simulink library "ee_lib_for_power_systems"  (R2025b)
settings: Solver=VariableStepAuto  StartTime=0.0  StopTime=10.0  RelTol=1e-3
undefined symbols (not defined in the file or in its folder): Alt_PolePair_Count, C3m, DiscIntNeverNeededParam, Enum1to12, L3m, Total_SM_Inertia, Ts
libraries: fl_lib x291, nesl_utility x219, ee_lib x139, custom_lib x12, $bdroot x10, ee_sl_lib x3, eeTransforms x2, eeGeneralControl x1, simulink x1
(part 8 of 11)

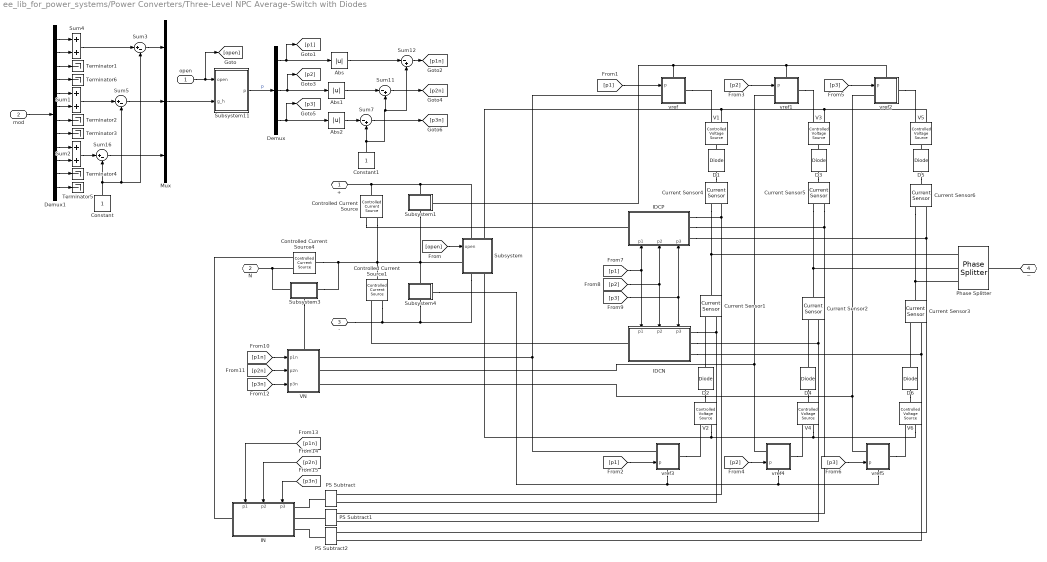
== system ee_lib_for_power_systems/Power Converters/Three-Level NPC Average-Switch with Diodes ==
  open : Inport
  mod : Inport  Port=2
  Abs : Abs  SaturateOnIntegerOverflow=off
  Abs1 : Abs  SaturateOnIntegerOverflow=off
  Abs2 : Abs  SaturateOnIntegerOverflow=off
  Constant : Constant  SampleTime=Ts
  Constant1 : Constant  SampleTime=Ts
  Controlled Current Source : fl_lib/Electrical/Electrical Sources/Controlled Current Source
  Controlled Current Source1 : fl_lib/Electrical/Electrical Sources/Controlled Current Source
  Controlled Current Source4 : fl_lib/Electrical/Electrical Sources/Controlled Current Source
  Current Sensor{1..6} (6 alike) : fl_lib/Electrical/Electrical Sensors/Current Sensor
  D{1..6} (6 alike) : fl_lib/Electrical/Electrical Elements/Diode  Vf=Vf V  Ron=Ron Ohm  Goff=Goff 1/Ohm  i_specify=off  i_priority=None  i=0 A  i_nominal_specify=off  i_nominal_value=1  i_nominal_unit=A  v_specify=off  v_priority=None  v=0 V  v_nominal_specify=off  v_nominal_value=1  v_nominal_unit=V
  Demux : Demux  Outputs=3
  Demux1 : Demux  Outputs=12
  IDCN : Subsystem
      settings: PortSchema="{\"entries\":[{\"content\":{\"sides\":[{\"content\":{\"connectorIds\":[\"LConn1\",\"LConn2\",\"LConn3\"]},\"type\":\"ConnectorPlacement.EquallySpacedRectSide\",\"uuid\":\"5fd9aafb-b495-44e2-9499-8cdfe2ab4f56\"},{\"content\":{\"connectorIds\":[\"RConn1\"],\"side\":\"RIGHT\"},\"type\":\"ConnectorPlacement.EquallySpacedRectSide\",\"uuid\":\"0d8ceae3-09aa-4016-97de-ee2249771a91\"},{\"content\":{\"connectorIds\":[\"In1\",\"In2\",\"In3\"],\"side\":\"TOP\"},\"type\":\"ConnectorPlacement.EquallySpacedRectSide\",\"uuid\":\"0b4c8ea8-b524-451d-8365-47bbe880e547\"},{\"content\":{\"side\":\"BOTTOM\"},\"type\":\"ConnectorPlacement.EquallySpacedRectSide\",\"uuid\":\"91c10802-5411-4afb-b6a0-ea8a924d9d4e\"}]},\"type\":\"ConnectorPlacement.EquallySpacedConnector\",\"uuid\":\"44c8dc65-a8bd-4e02-863f-9a87b0ac0f96\"}],\"packageUris\":[\"http://schema.mathworks.com/mf0/Connector/R2020b\"],\"version\":\"1.0\"}"
  IDCP : Subsystem
      settings: PortSchema="{\"entries\":[{\"content\":{\"sides\":[{\"content\":{\"connectorIds\":[\"LConn1\",\"LConn2\",\"LConn3\"]},\"type\":\"ConnectorPlacement.EquallySpacedRectSide\",\"uuid\":\"5fd9aafb-b495-44e2-9499-8cdfe2ab4f56\"},{\"content\":{\"connectorIds\":[\"RConn1\"],\"side\":\"RIGHT\"},\"type\":\"ConnectorPlacement.EquallySpacedRectSide\",\"uuid\":\"0d8ceae3-09aa-4016-97de-ee2249771a91\"},{\"content\":{\"side\":\"TOP\"},\"type\":\"ConnectorPlacement.EquallySpacedRectSide\",\"uuid\":\"0b4c8ea8-b524-451d-8365-47bbe880e547\"},{\"content\":{\"connectorIds\":[\"In1\",\"In2\",\"In3\"],\"side\":\"BOTTOM\"},\"type\":\"ConnectorPlacement.EquallySpacedRectSide\",\"uuid\":\"91c10802-5411-4afb-b6a0-ea8a924d9d4e\"}]},\"type\":\"ConnectorPlacement.EquallySpacedConnector\",\"uuid\":\"44c8dc65-a8bd-4e02-863f-9a87b0ac0f96\"}],\"packageUris\":[\"http://schema.mathworks.com/mf0/Connector/R2020b\"],\"version\":\"1.0\"}"
  IN : Subsystem
      settings: PortSchema="{\"entries\":[{\"content\":{\"sides\":[{\"content\":{\"connectorIds\":[\"LConn1\",\"LConn2\",\"LConn3\"]},\"type\":\"ConnectorPlacement.EquallySpacedRectSide\",\"uuid\":\"5fd9aafb-b495-44e2-9499-8cdfe2ab4f56\"},{\"content\":{\"connectorIds\":[\"RConn1\"],\"side\":\"RIGHT\"},\"type\":\"ConnectorPlacement.EquallySpacedRectSide\",\"uuid\":\"0d8ceae3-09aa-4016-97de-ee2249771a91\"},{\"content\":{\"connectorIds\":[\"In1\",\"In2\",\"In3\"],\"side\":\"TOP\"},\"type\":\"ConnectorPlacement.EquallySpacedRectSide\",\"uuid\":\"0b4c8ea8-b524-451d-8365-47bbe880e547\"},{\"content\":{\"side\":\"BOTTOM\"},\"type\":\"ConnectorPlacement.EquallySpacedRectSide\",\"uuid\":\"91c10802-5411-4afb-b6a0-ea8a924d9d4e\"}]},\"type\":\"ConnectorPlacement.EquallySpacedConnector\",\"uuid\":\"44c8dc65-a8bd-4e02-863f-9a87b0ac0f96\"}],\"packageUris\":[\"http://schema.mathworks.com/mf0/Connector/R2020b\"],\"version\":\"1.0\"}"
  Mux : Mux  Inputs=3
  PS Subtract : fl_lib/Physical Signals/Functions/PS Subtract
  PS Subtract1 : fl_lib/Physical Signals/Functions/PS Subtract
  PS Subtract2 : fl_lib/Physical Signals/Functions/PS Subtract
  Phase Splitter : ee_lib/Connectors & References/Phase Splitter
  Subsystem : Subsystem
      settings: PortSchema="{\"entries\":[{\"content\":{\"sides\":[{\"content\":{\"connectorIds\":[\"In1\",\"LConn1\"]},\"type\":\"ConnectorPlacement.EquallySpacedRectSide\",\"uuid\":\"88db1bd8-f64b-4165-8d2c-7cfa4014cba2\"},{\"content\":{\"side\":\"RIGHT\"},\"type\":\"ConnectorPlacement.EquallySpacedRectSide\",\"uuid\":\"19f0c499-9eed-48d3-a8f5-fe9dad581b85\"},{\"content\":{\"connectorIds\":[\"LConn2\",\"LConn3\"],\"side\":\"TOP\"},\"type\":\"ConnectorPlacement.EquallySpacedRectSide\",\"uuid\":\"71d5fe89-ffc2-4430-819d-512bfd197a1c\"},{\"content\":{\"connectorIds\":[\"RConn1\",\"RConn2\"],\"side\":\"BOTTOM\"},\"type\":\"ConnectorPlacement.EquallySpacedRectSide\",\"uuid\":\"b828cb58-5f78-4b23-b8a0-147c7a9d81e7\"}]},\"type\":\"ConnectorPlacement.EquallySpacedConnector\",\"uuid\":\"64b174d4-80a0-4eb7-9a75-e3677787263f\"}],\"packageUris\":[\"http://schema.mathworks.com/mf0/Connector/R2020b\"],\"version\":\"1.0\"}"
  Subsystem1 : Subsystem
      settings: PortSchema="{\"entries\":[{\"content\":{\"sides\":[{\"content\":{\"connectorIds\":[\"LConn1\"]},\"type\":\"ConnectorPlacement.EquallySpacedRectSide\",\"uuid\":\"657010cc-ebac-44a1-b1fa-70bc77d723fd\"},{\"content\":{\"connectorIds\":[\"RConn1\"],\"side\":\"RIGHT\"},\"type\":\"ConnectorPlacement.EquallySpacedRectSide\",\"uuid\":\"4e06f12d-4c30-449d-8090-9eefcb844bcd\"},{\"content\":{\"side\":\"TOP\"},\"type\":\"ConnectorPlacement.EquallySpacedRectSide\",\"uuid\":\"366a080c-b244-4111-b523-cca255cda3b4\"},{\"content\":{\"connectorIds\":[\"RConn2\"],\"side\":\"BOTTOM\"},\"type\":\"ConnectorPlacement.EquallySpacedRectSide\",\"uuid\":\"d10f8831-4450-471d-a877-48a3f6294e5e\"}]},\"type\":\"ConnectorPlacement.EquallySpacedConnector\",\"uuid\":\"eaac196a-9b15-4081-b7ac-14663ddf5688\"}],\"packageUris\":[\"http://schema.mathworks.com/mf0/Connector/R2020b\"],\"version\":\"1.0\"}"
  Subsystem11 : Subsystem
  Subsystem3 : Subsystem
      settings: PortSchema="{\"entries\":[{\"content\":{\"sides\":[{\"content\":{\"connectorIds\":[\"LConn1\"]},\"type\":\"ConnectorPlacement.EquallySpacedRectSide\",\"uuid\":\"657010cc-ebac-44a1-b1fa-70bc77d723fd\"},{\"content\":{\"connectorIds\":[\"RConn1\"],\"side\":\"RIGHT\"},\"type\":\"ConnectorPlacement.EquallySpacedRectSide\",\"uuid\":\"4e06f12d-4c30-449d-8090-9eefcb844bcd\"},{\"content\":{\"connectorIds\":[\"LConn2\"],\"side\":\"TOP\"},\"type\":\"ConnectorPlacement.EquallySpacedRectSide\",\"uuid\":\"366a080c-b244-4111-b523-cca255cda3b4\"},{\"content\":{\"side\":\"BOTTOM\"},\"type\":\"ConnectorPlacement.EquallySpacedRectSide\",\"uuid\":\"d10f8831-4450-471d-a877-48a3f6294e5e\"}]},\"type\":\"ConnectorPlacement.EquallySpacedConnector\",\"uuid\":\"eaac196a-9b15-4081-b7ac-14663ddf5688\"}],\"packageUris\":[\"http://schema.mathworks.com/mf0/Connector/R2020b\"],\"version\":\"1.0\"}"
  Subsystem4 : Subsystem
      settings: PortSchema="{\"entries\":[{\"content\":{\"sides\":[{\"content\":{\"connectorIds\":[\"LConn1\"]},\"type\":\"ConnectorPlacement.EquallySpacedRectSide\",\"uuid\":\"657010cc-ebac-44a1-b1fa-70bc77d723fd\"},{\"content\":{\"connectorIds\":[\"RConn1\"],\"side\":\"RIGHT\"},\"type\":\"ConnectorPlacement.EquallySpacedRectSide\",\"uuid\":\"4e06f12d-4c30-449d-8090-9eefcb844bcd\"},{\"content\":{\"side\":\"TOP\"},\"type\":\"ConnectorPlacement.EquallySpacedRectSide\",\"uuid\":\"366a080c-b244-4111-b523-cca255cda3b4\"},{\"content\":{\"connectorIds\":[\"RConn2\"],\"side\":\"BOTTOM\"},\"type\":\"ConnectorPlacement.EquallySpacedRectSide\",\"uuid\":\"d10f8831-4450-471d-a877-48a3f6294e5e\"}]},\"type\":\"ConnectorPlacement.EquallySpacedConnector\",\"uuid\":\"eaac196a-9b15-4081-b7ac-14663ddf5688\"}],\"packageUris\":[\"http://schema.mathworks.com/mf0/Connector/R2020b\"],\"version\":\"1.0\"}"
  Sum1 : Sum
  Sum11 : Sum  Inputs=|-+
  Sum12 : Sum  Inputs=|-+
  Sum16 : Sum  Inputs=|+-
  Sum2 : Sum
  Sum3 : Sum  Inputs=|+-
  Sum4 : Sum
  Sum5 : Sum  Inputs=|+-
  Sum7 : Sum  Inputs=|-+
  Terminator{1..6} (6 alike) : Terminator
  V{1..6} (6 alike) : fl_lib/Electrical/Electrical Sources/Controlled Voltage Source
  VN : Subsystem
      settings: PortSchema="{\"entries\":[{\"content\":{\"sides\":[{\"content\":{\"connectorIds\":[\"In1\",\"In2\",\"In3\"]},\"type\":\"ConnectorPlacement.EquallySpacedRectSide\",\"uuid\":\"76446245-3b58-4011-85fd-f27f8ae281c8\"},{\"content\":{\"connectorIds\":[\"RConn1\",\"RConn2\",\"RConn3\"],\"side\":\"RIGHT\"},\"type\":\"ConnectorPlacement.EquallySpacedRectSide\",\"uuid\":\"0729b849-5916-4866-a3f0-df0ddf2f6c39\"},{\"content\":{\"connectorIds\":[\"LConn1\"],\"side\":\"TOP\"},\"type\":\"ConnectorPlacement.EquallySpacedRectSide\",\"uuid\":\"732bc8a4-0f08-4f07-8caa-b1f4c499d2f5\"}]},\"type\":\"ConnectorPlacement.EquallySpacedConnector\",\"uuid\":\"056633d8-005f-41b2-9737-b2dcd7b802cb\"}],\"packageUris\":[\"http://schema.mathworks.com/mf0/Connector/R2020b\"],\"version\":\"1.0\"}"
  vref : Subsystem
      settings: PortSchema="{\"entries\":[{\"content\":{\"sides\":[{\"content\":{\"connectorIds\":[\"In1\",\"LConn1\"]},\"type\":\"ConnectorPlacement.EquallySpacedRectSide\",\"uuid\":\"177b0f26-b09e-4dc8-aee9-3547e6e38c36\"},{\"content\":{\"connectorIds\":[\"RConn1\"],\"side\":\"RIGHT\"},\"type\":\"ConnectorPlacement.EquallySpacedRectSide\",\"uuid\":\"51253abf-338b-44bf-b091-8aed177abf09\"},{\"content\":{\"connectorIds\":[\"LConn2\"],\"side\":\"TOP\"},\"type\":\"ConnectorPlacement.EquallySpacedRectSide\",\"uuid\":\"62148f0b-b6fd-4066-b7ab-63aa80b90d1b\"}]},\"type\":\"ConnectorPlacement.EquallySpacedConnector\",\"uuid\":\"16fdcc32-ca93-497b-869b-72fbbd5f95c5\"}],\"packageUris\":[\"http://schema.mathworks.com/mf0/Connector/R2020b\"],\"version\":\"1.0\"}"
  vref1 : Subsystem
      settings: PortSchema="{\"entries\":[{\"content\":{\"sides\":[{\"content\":{\"connectorIds\":[\"In1\",\"LConn1\"]},\"type\":\"ConnectorPlacement.EquallySpacedRectSide\",\"uuid\":\"177b0f26-b09e-4dc8-aee9-3547e6e38c36\"},{\"content\":{\"connectorIds\":[\"RConn1\"],\"side\":\"RIGHT\"},\"type\":\"ConnectorPlacement.EquallySpacedRectSide\",\"uuid\":\"51253abf-338b-44bf-b091-8aed177abf09\"},{\"content\":{\"connectorIds\":[\"LConn2\"],\"side\":\"TOP\"},\"type\":\"ConnectorPlacement.EquallySpacedRectSide\",\"uuid\":\"62148f0b-b6fd-4066-b7ab-63aa80b90d1b\"}]},\"type\":\"ConnectorPlacement.EquallySpacedConnector\",\"uuid\":\"16fdcc32-ca93-497b-869b-72fbbd5f95c5\"}],\"packageUris\":[\"http://schema.mathworks.com/mf0/Connector/R2020b\"],\"version\":\"1.0\"}"
  vref2 : Subsystem
      settings: PortSchema="{\"entries\":[{\"content\":{\"sides\":[{\"content\":{\"connectorIds\":[\"In1\",\"LConn1\"]},\"type\":\"ConnectorPlacement.EquallySpacedRectSide\",\"uuid\":\"177b0f26-b09e-4dc8-aee9-3547e6e38c36\"},{\"content\":{\"connectorIds\":[\"RConn1\"],\"side\":\"RIGHT\"},\"type\":\"ConnectorPlacement.EquallySpacedRectSide\",\"uuid\":\"51253abf-338b-44bf-b091-8aed177abf09\"},{\"content\":{\"connectorIds\":[\"LConn2\"],\"side\":\"TOP\"},\"type\":\"ConnectorPlacement.EquallySpacedRectSide\",\"uuid\":\"62148f0b-b6fd-4066-b7ab-63aa80b90d1b\"}]},\"type\":\"ConnectorPlacement.EquallySpacedConnector\",\"uuid\":\"16fdcc32-ca93-497b-869b-72fbbd5f95c5\"}],\"packageUris\":[\"http://schema.mathworks.com/mf0/Connector/R2020b\"],\"version\":\"1.0\"}"
  vref3 : Subsystem
      settings: PortSchema="{\"entries\":[{\"content\":{\"sides\":[{\"content\":{\"connectorIds\":[\"LConn1\",\"In1\"]},\"type\":\"ConnectorPlacement.EquallySpacedRectSide\",\"uuid\":\"177b0f26-b09e-4dc8-aee9-3547e6e38c36\"},{\"content\":{\"connectorIds\":[\"RConn1\"],\"side\":\"RIGHT\"},\"type\":\"ConnectorPlacement.EquallySpacedRectSide\",\"uuid\":\"51253abf-338b-44bf-b091-8aed177abf09\"},{\"content\":{\"side\":\"TOP\"},\"type\":\"ConnectorPlacement.EquallySpacedRectSide\",\"uuid\":\"62148f0b-b6fd-4066-b7ab-63aa80b90d1b\"},{\"content\":{\"connectorIds\":[\"RConn2\"],\"side\":\"BOTTOM\"},\"type\":\"ConnectorPlacement.EquallySpacedRectSide\",\"uuid\":\"f032becc-e905-4cd9-aa70-19abd7f3ae13\"}]},\"type\":\"ConnectorPlacement.EquallySpacedConnector\",\"uuid\":\"16fdcc32-ca93-497b-869b-72fbbd5f95c5\"}],\"packageUris\":[\"http://schema.mathworks.com/mf0/Connector/R2020b\"],\"version\":\"1.0\"}"
  vref4 : Subsystem
      settings: PortSchema="{\"entries\":[{\"content\":{\"sides\":[{\"content\":{\"connectorIds\":[\"LConn1\",\"In1\"]},\"type\":\"ConnectorPlacement.EquallySpacedRectSide\",\"uuid\":\"177b0f26-b09e-4dc8-aee9-3547e6e38c36\"},{\"content\":{\"connectorIds\":[\"RConn1\"],\"side\":\"RIGHT\"},\"type\":\"ConnectorPlacement.EquallySpacedRectSide\",\"uuid\":\"51253abf-338b-44bf-b091-8aed177abf09\"},{\"content\":{\"side\":\"TOP\"},\"type\":\"ConnectorPlacement.EquallySpacedRectSide\",\"uuid\":\"62148f0b-b6fd-4066-b7ab-63aa80b90d1b\"},{\"content\":{\"connectorIds\":[\"RConn2\"],\"side\":\"BOTTOM\"},\"type\":\"ConnectorPlacement.EquallySpacedRectSide\",\"uuid\":\"f032becc-e905-4cd9-aa70-19abd7f3ae13\"}]},\"type\":\"ConnectorPlacement.EquallySpacedConnector\",\"uuid\":\"16fdcc32-ca93-497b-869b-72fbbd5f95c5\"}],\"packageUris\":[\"http://schema.mathworks.com/mf0/Connector/R2020b\"],\"version\":\"1.0\"}"
  vref5 : Subsystem
      settings: PortSchema="{\"entries\":[{\"content\":{\"sides\":[{\"content\":{\"connectorIds\":[\"LConn1\",\"In1\"]},\"type\":\"ConnectorPlacement.EquallySpacedRectSide\",\"uuid\":\"177b0f26-b09e-4dc8-aee9-3547e6e38c36\"},{\"content\":{\"connectorIds\":[\"RConn1\"],\"side\":\"RIGHT\"},\"type\":\"ConnectorPlacement.EquallySpacedRectSide\",\"uuid\":\"51253abf-338b-44bf-b091-8aed177abf09\"},{\"content\":{\"side\":\"TOP\"},\"type\":\"ConnectorPlacement.EquallySpacedRectSide\",\"uuid\":\"62148f0b-b6fd-4066-b7ab-63aa80b90d1b\"},{\"content\":{\"connectorIds\":[\"RConn2\"],\"side\":\"BOTTOM\"},\"type\":\"ConnectorPlacement.EquallySpacedRectSide\",\"uuid\":\"f032becc-e905-4cd9-aa70-19abd7f3ae13\"}]},\"type\":\"ConnectorPlacement.EquallySpacedConnector\",\"uuid\":\"16fdcc32-ca93-497b-869b-72fbbd5f95c5\"}],\"packageUris\":[\"http://schema.mathworks.com/mf0/Connector/R2020b\"],\"version\":\"1.0\"}"
  + : PMIOPort  Side=Left
  N : PMIOPort  Port=2  Side=Left
  - : PMIOPort  Port=3  Side=Left
  ~ : PMIOPort  Port=4  Side=Right
  wires:
  [p3] -> IDCP/1(p3), IDCN/1(p3)
  [p1] -> IDCP/3(p1), IDCN/3(p1)
  Abs2/1 -> Sum7/1
  Abs1/1 -> Sum11/1
  Abs/1 -> Sum12/1
  Constant1/1 -> Sum12/2, Sum11/2, Sum7/2
  Demux/3 -> Abs2/1, [p3]
  Demux/2 -> Abs1/1, [p2]
  Demux1/6 -> Sum1/2
  Sum3/1 -> Mux/1
  Demux1/10 -> Sum2/2
  Demux1/2 -> Sum4/2
  Demux1/9 -> Sum2/1
  Demux1/5 -> Sum1/1
  Sum4/1 -> Sum3/1
  Demux1/1 -> Sum4/1
  [p2] -> vref4/1(p)
  mod/1 -> Demux1/1
  Sum1/1 -> Sum5/1
  Sum2/1 -> Sum16/1
  Sum16/1 -> Mux/3
  Constant/1 -> Sum3/2, Sum5/2, Sum16/2
  [p2] -> IDCP/2(p2), IDCN/2(p2)
  Demux1/7 -> Terminator2/1
  Demux1/8 -> Terminator3/1
  Demux1/11 -> Terminator4/1
  Demux1/12 -> Terminator5/1
  Demux1/3 -> Terminator1/1
  Demux1/4 -> Terminator6/1
  Sum5/1 -> Mux/2
  Demux/1 -> Abs/1, [p1]
  Mux/1 -> Subsystem11/2(g_h)
  Subsystem11/1(p) -> Demux/1  "p"
  open/1 -> Subsystem11/1(open), [open]
  [open] -> Subsystem/1(open)
  Sum12/1 -> [p1n]
  Sum11/1 -> [p2n]
  Sum7/1 -> [p3n]
  [p1n] -> IN/3(p1)
  [p2n] -> IN/2(p2)
  [p3n] -> IN/1(p3)
  [p1] -> vref/1(p)
  [p2] -> vref1/1(p)
  [p3] -> vref2/1(p)
  [p1n] -> VN/1(p1n)
  [p2n] -> VN/2(p2n)
  [p3n] -> VN/3(p3n)
  [p1] -> vref3/1(p)
  [p3] -> vref5/1(p)
  connections:
  D5/RConn1 -- V5/LConn1
  D3/RConn1 -- V3/LConn1
  IDCN/LConn3 -- Current Sensor3/RConn1 -- PS Subtract2/LConn2
  IDCN/LConn2 -- Current Sensor2/RConn1 -- PS Subtract1/LConn2
  IDCN/LConn1 -- Current Sensor1/RConn1 -- PS Subtract/LConn2
  D6/RConn1 -- Current Sensor3/RConn2
  D6/LConn1 -- V6/LConn1
  D4/LConn1 -- V4/LConn1
  D4/RConn1 -- Current Sensor2/RConn2
  Current Sensor4/RConn2 -- Phase Splitter/RConn1 -- Current Sensor1/LConn1
  D2/LConn1 -- V2/LConn1
  Current Sensor4/RConn1 -- PS Subtract/LConn1 -- IDCP/LConn1
  Current Sensor5/RConn1 -- IDCP/LConn2 -- PS Subtract1/LConn1
  IDCP/LConn3 -- Current Sensor6/RConn1 -- PS Subtract2/LConn1
  ~/RConn1 -- Phase Splitter/LConn1
  Current Sensor6/RConn2 -- Phase Splitter/RConn3 -- Current Sensor3/LConn1
  Subsystem/LConn2 -- Subsystem1/LConn1 -- Controlled Current Source/LConn1 -- +/RConn1
  Controlled Current Source/RConn1 -- IDCP/RConn1
  IDCN/RConn1 -- Controlled Current Source1/RConn1
  Subsystem4/RConn2 -- vref5/RConn2 -- vref4/RConn2 -- vref3/RConn2
  D2/RConn1 -- Current Sensor1/RConn2
  vref/LConn2 -- Subsystem1/RConn2 -- vref2/LConn2 -- vref1/LConn2
  Controlled Current Source4/RConn2 -- Subsystem3/LConn1 -- N/RConn1
  V1/RConn2 -- Subsystem/LConn3 -- V3/RConn2 -- V5/RConn2
  D1/RConn1 -- V1/LConn1
  Controlled Current Source1/RConn2 -- -/RConn1 -- Subsystem/RConn1 -- Subsystem4/RConn1
  D1/LConn1 -- Current Sensor4/LConn1
  D3/LConn1 -- Current Sensor5/LConn1
  D5/LConn1 -- Current Sensor6/LConn1
  Current Sensor5/RConn2 -- Phase Splitter/RConn2 -- Current Sensor2/LConn1
  Controlled Current Source4/LConn1 -- Subsystem3/RConn1 -- Controlled Current Source/RConn2 -- Controlled Current Source1/LConn1 -- Subsystem4/LConn1 -- Subsystem1/RConn1 -- Subsystem/LConn1
  vref1/RConn1 -- V3/RConn1
  vref/RConn1 -- V1/RConn1
  vref2/RConn1 -- V5/RConn1
  vref/LConn1 -- vref3/LConn1 -- VN/RConn1
  vref3/RConn1 -- V2/RConn1
  vref4/RConn1 -- V4/RConn1
  VN/RConn2 -- vref4/LConn1 -- vref1/LConn1
  vref5/RConn1 -- V6/RConn1
  VN/RConn3 -- vref5/LConn1 -- vref2/LConn1
  IN/RConn1 -- Controlled Current Source4/RConn1
  PS Subtract/RConn1 -- IN/LConn1
  PS Subtract1/RConn1 -- IN/LConn2
  PS Subtract2/RConn1 -- IN/LConn3
  V6/RConn2 -- V2/RConn2 -- Subsystem/RConn2 -- V4/RConn2
  Subsystem3/LConn2 -- VN/LConn1

== system ee_lib_for_power_systems/Power Converters/Thyristor ==
  g : Inport
  Simulink-PS Converter1 : nesl_utility/Simulink-PS Converter  PhysicalDomain=network_engine_domain  SubClassName=ps_input  LeftPortType=input  RightPortType=output  PseudoPeriodic=off  Frequencies=[]  NoiseDistribution=none  NoiseParameters=[]  Unit=1  AffineConversion=off  FilteringAndDerivatives=zero  SimscapeFilterOrder=1  InputFilterTimeConstant=0.001  UdotUserProvided=0
  thyristor : ee_lib/Semiconductors & Converters/Thyristor (Piecewise Linear)  port_option=ee.enum.controlport.ps  Vf=Vf V  Ron=Ron Ohm  Goff=Goff 1/Ohm  Vgt=0.5 V  Ih=1e-3 A  diode_param=ee.enum.converters.protectiondiode.none  diode_Vf=0.8 V  diode_Ron=0.001 Ohm  diode_Goff=1e-5 1/Ohm  diode_C=50e-9 F  diode_iRM=-235 A  diode_iF=300 A  diode_idot=-50 A/us  diode_trr_param=ee.enum.converters.diodetrr.trr_factor  diode_trr_factor=3  diode_trr=15 us  diode_Qrr=1500 s*uA  iak_specify=off  iak_priority=None  iak=0 A  iak_nominal_specify=off  iak_nominal_value=1  iak_nominal_unit=A  vak_specify=off  vak_priority=None  vak=0 V  vak_nominal_specify=off  vak_nominal_value=1  vak_nominal_unit=V  vgk_specify=off  vgk_priority=None  vgk=0 V  vgk_nominal_specify=off  vgk_nominal_value=1  vgk_nominal_unit=V  thermal_loss_option=ee.enum.converters.thermalLossOption.constantvalues  Von_data=[0, .1, .6, .8, 1, 1.3, 1.6, 2, 2.4; 0, .1, .7, 1, 1.2, 1.5, 1.9, 2.4, 2.8] V  Tj=[298.15, 398.15] K  Iout_data=[0, .1, 1, 5, 10, 20, 40, 70, 100] A  Eon_data=[0, .0024, .024, .12, .2, .48, 1.04, 2.16, 3.24; 0, .003, .03, .15, .25, .6, 1.3, 2.7, 4.05] * 1e-3 J  Tj_loss=[298.15, 398.15] K  Iout_data_loss=[0, .1, 1, 5, 10, 20, 40, 70, 100] A  Eon_scalar=22.86e-3 J  Eoff_natural_commutation=10e-3 J  Voff=300 V  Iout_scalar=600 A  onCurrentMeasDelay=1e-4 s  diodeModelType=ee.enum.converters.diodeModelType.pwl  diodeTableType=ee.enum.diode.tableType.IfMat  diode_IfMat=[.07, .12, .19, 1.75, 4.24, 7.32, 11.2; .16, .3, .72, 2.14, 4.02, 6.35, 9.12] A  diode_VfMat=[.9, 1.15, 1.25, 1.5, 1.75, 2.17, 2.6, 2.85; .58, .68, .75, 1.1, 1.38, 1.77, 2.27, 2.7] V  diode_TjVec=[25, 125] degC  diode_VfVec=[.5, .7, .9, 1.3, 1.7, 2.1, 2.5] V  diode_IfVec=[.1, .2, .5, 1, 2, 4, 7, 10] A  diode_rec_loss_model=ee.enum.diode.reverseRecoveryModel.fixed  diode_ErecVal=0 J  diode_scaleWithIandV=true  diode_ErecMat=zeros(2, 3) J  diode_TjrecVec=[298.15, 398.15] K  diode_IfrecVec=[.1, 1, 10] A  diode_IrecVal=10 A  diode_VrecVal=10 V  diode_tFilter=1 / 1000 / 100 s  thermal_network_parameterization=ee.enum.thermalNetworkTopology.external  Rth=[.08, .5] K/W  Rth_Cauer=[.08, .1, .5] K/W  Rth_Foster=[.08, .14, .22, .16] K/W  thermal_mass_parameterization=ee.enum.thermalNetwork.timeconstant  thermal_mass=[.01, .5] J/K  thermal_mass_Cauer=[.01, .1, .5] J/K  thermal_mass_Foster=[.001, .005, .05, .5] J/K  thermal_time_constant=[.001, .2] s  thermal_time_constant_Cauer=[.001, .1, .2] s  thermal_time_constant_Foster=[7e-05, .0007, .01, .08] s  thermal_mass_initial_temperatures=[25, 25] degC  thermal_mass_initial_temperatures_Cauer=[25, 25, 25] degC  junction_thermal_mass=0.01 J/K  Tvec_initial_Foster=[25, 25, 25, 25] degC  junction_temperature_specify=off  junction_temperature_priority=None  junction_temperature=25 degC  junction_temperature_nominal_specify=off  junction_temperature_nominal_value=1  junction_temperature_nominal_unit=degC  Qj_specify=off  Qj_priority=None  Qj=0 W  Qj_nominal_specify=off  Qj_nominal_value=1  Qj_nominal_unit=W  accumulatedSwitchingLosses_specify=off  accumulatedSwitchingLosses_priority=None  accumulatedSwitchingLosses=0 J
      settings: InternalSimscapePortConfiguration="{\"Bottom\":[],\"Left\":[{\"id\":\"G\",\"label\":\"\",\"type\":\"input\"}],\"Right\":[{\"id\":\"A\",\"label\":\"\",\"type\":\"foundation.electrical.electrical\"},{\"id\":\"K\",\"label\":\"\",\"type\":\"foundation.electrical.electrical\"}],\"Top\":[]}"
  a : PMIOPort  Side=Left
  k : PMIOPort  Port=2  Side=Right
  wires:
  g/1 -> Simulink-PS Converter1/1
  connections:
  thyristor/LConn1 -- Simulink-PS Converter1/RConn1
  thyristor/RConn1 -- a/RConn1
  thyristor/RConn2 -- k/RConn1

== system ee_lib_for_power_systems/Power Converters/Thyristor Bridge (Ideal) ==
  g : Inport
  Demux : Demux  Outputs=6
  Phase Splitter : ee_lib/Connectors & References/Phase Splitter
  S1 : Subsystem
      settings: PortSchema="{\"entries\":[{\"content\":{\"sides\":[{\"content\":{\"connectorIds\":[\"LConn1\"]},\"type\":\"ConnectorPlacement.EquallySpacedRectSide\",\"uuid\":\"022a574f-444f-453c-a1d1-ee0757b89aa3\"},{\"content\":{\"connectorIds\":[\"RConn1\"],\"side\":\"RIGHT\"},\"type\":\"ConnectorPlacement.EquallySpacedRectSide\",\"uuid\":\"24ba200a-3584-4d80-b199-fea7992bc5cd\"},{\"content\":{\"side\":\"TOP\"},\"type\":\"ConnectorPlacement.EquallySpacedRectSide\",\"uuid\":\"c00da2b4-4d59-4de1-bdfc-8f040e3cf978\"},{\"content\":{\"connectorIds\":[\"In1\"],\"side\":\"BOTTOM\"},\"type\":\"ConnectorPlacement.EquallySpacedRectSide\",\"uuid\":\"5313685b-0d93-477b-a1b7-9a9dcc5834c8\"}]},\"type\":\"ConnectorPlacement.EquallySpacedConnector\",\"uuid\":\"3c73b747-5965-477a-97a9-fd3b811917db\"}],\"packageUris\":[\"http://schema.mathworks.com/mf0/Connector/R2020b\"],\"version\":\"1.0\"}"
  S{2..6} (5 alike) : $bdroot/Power Converters/Thyristor Bridge (Ideal)/S1  SystemSampleTime=-1  VariantActivationTime=update diagram  EmptyChoice=off  PassThrough=off  PassThroughVariantControl=(default)  EmptyChoiceVariantControl=(default)  AllowFlexibleInterface=on  Latency=0  AutoFrameSizeCalculation=off
      settings: PortSchema="{\"entries\":[{\"content\":{\"sides\":[{\"content\":{\"connectorIds\":[\"LConn1\"]},\"type\":\"ConnectorPlacement.EquallySpacedRectSide\",\"uuid\":\"022a574f-444f-453c-a1d1-ee0757b89aa3\"},{\"content\":{\"connectorIds\":[\"RConn1\"],\"side\":\"RIGHT\"},\"type\":\"ConnectorPlacement.EquallySpacedRectSide\",\"uuid\":\"24ba200a-3584-4d80-b199-fea7992bc5cd\"},{\"content\":{\"side\":\"TOP\"},\"type\":\"ConnectorPlacement.EquallySpacedRectSide\",\"uuid\":\"c00da2b4-4d59-4de1-bdfc-8f040e3cf978\"},{\"content\":{\"connectorIds\":[\"In1\"],\"side\":\"BOTTOM\"},\"type\":\"ConnectorPlacement.EquallySpacedRectSide\",\"uuid\":\"5313685b-0d93-477b-a1b7-9a9dcc5834c8\"}]},\"type\":\"ConnectorPlacement.EquallySpacedConnector\",\"uuid\":\"3c73b747-5965-477a-97a9-fd3b811917db\"}],\"packageUris\":[\"http://schema.mathworks.com/mf0/Connector/R2020b\"],\"version\":\"1.0\"}"
  ~ : PMIOPort  Side=Left
  + : PMIOPort  Port=2  Side=Right
  - : PMIOPort  Port=3  Side=Right
  wires:
  g/1 -> Demux/1
  [g3] -> S3/1
  [g6] -> S6/1
  [g2] -> S2/1
  [g4] -> S4/1
  [g5] -> S5/1
  [g1] -> S1/1(G)
  Demux/1 -> [g1]
  Demux/2 -> [g2]
  Demux/6 -> [g6]
  Demux/5 -> [g5]
  Demux/4 -> [g4]
  Demux/3 -> [g3]
  connections:
  S2/RConn1 -- -/RConn1 -- S6/RConn1 -- S4/RConn1
  S1/LConn1 -- S3/LConn1 -- S5/LConn1 -- +/RConn1
  Phase Splitter/RConn2 -- S6/LConn1 -- S3/RConn1
  S1/RConn1 -- S4/LConn1 -- Phase Splitter/RConn1
  Phase Splitter/RConn3 -- S5/RConn1 -- S2/LConn1
  ~/RConn1 -- Phase Splitter/LConn1
